simulink model "MCVT_Integration"  (R2020a)
settings: Solver=ode8  StartTime=0.0  StopTime=T_end  FixedStep=dt  RelTol=1e-3  EnableMultiTasking=on
values from neighbouring files (symbols this model uses):
  assumed_power_voltage = 1.25e2    (= 125)    [MCVT_Input_File.m]    (also in MCVT_Run_QuickCheck.m)
  battery_power_toggle = 1    [MCVT_Input_File.m]    (also in MCVT_Run_QuickCheck.m)
  battery_trickle_charge_param = 0.1    [MCVT_Input_File.m]    (also in MCVT_Run_QuickCheck.m)
  battery_trickle_leakage_param = 0.01    [MCVT_Input_File.m]    (also in MCVT_Run_QuickCheck.m)
  damage_case_id = 3    [MCVT_Input_File.m]    (also in MCVT_Run_QuickCheck.m = 1)
  dt = 1/fs    (= 0.001)    [MCVT_Run_QuickCheck.m]    (also in MCVT_Sim_Set.m)
  dt_2 = 1/fs_2    (= 0.001)    [MCVT_Run_QuickCheck.m]    (also in MCVT_Sim_Set.m)
  dt_3 = 1/fs_3    (= 0.001)    [MCVT_Run_QuickCheck.m]    (also in MCVT_Sim_Set.m)
  dt_5 = 1/fs_5    (= 0.005)    [MCVT_Run_QuickCheck.m]    (also in MCVT_Sim_Set.m)
  dt_8 = 1/fs_8    (= 0.05)    [MCVT_Run_QuickCheck.m]    (also in MCVT_Sim_Set.m)
  dt_9 = 1/fs_9    (= 0.001)    [MCVT_Run_QuickCheck.m]    (also in MCVT_Sim_Set.m)
  dust_met_duration = 3    [MCVT_Input_File.m]    (also in MCVT_Run_QuickCheck.m = 2)
  ECLSS_indoor_set_temperature = 298.15    [MCVT_Input_File.m]    (also in MCVT_Run_QuickCheck.m)
  energy_store_max_o = 150    [MCVT_Input_File.m]    (also in MCVT_Run_QuickCheck.m)
  energy_store_o = 120    [MCVT_Input_File.m]    (also in MCVT_Run_QuickCheck.m)
  fs = 1000    [MCVT_Run_QuickCheck.m]    (also in MCVT_Sim_Set.m)
  gain_charge_param = 1    [MCVT_Input_File.m]    (also in MCVT_Run_QuickCheck.m)
  ground_temperature = 373    [MCVT_Habitat_Design_Input_File.m]    (also in MCVT_Run_QuickCheck.m)
  I_max_charge = 800    [MCVT_Input_File.m]    (also in MCVT_Run_QuickCheck.m)
  I_max_discharge = 800    [MCVT_Input_File.m]    (also in MCVT_Run_QuickCheck.m)
  initial_pressure = 1e5    (= 100000)    [MCVT_Input_File.m]    (also in MCVT_Run_QuickCheck.m)
  initial_temperature = 295    [MCVT_Input_File.m]    (also in MCVT_Run_QuickCheck.m)
  interior_leak_rate = 0.00415    [MCVT_Input_File.m]    (also in MCVT_Run_QuickCheck.m)
  launch_dust_accum_start_time = 10    [MCVT_Input_File.m]    (also in MCVT_Run_QuickCheck.m = 2)
  launch_dust_accum_stop_time = 12    [MCVT_Input_File.m]    (also in MCVT_Run_QuickCheck.m = 3)
  meteorite_impact_time = 2    [MCVT_Input_File.m]    (also in MCVT_Run_QuickCheck.m = 1)
  nuclear_power_toggle = 1    [MCVT_Input_File.m]    (also in MCVT_Run_QuickCheck.m)
  print_interval = 30    [MCVT_Input_File.m]
  SOC_lower_thres = 0.5    [MCVT_Input_File.m]    (also in MCVT_Run_QuickCheck.m)
  SOC_upper_thres = 0.9    [MCVT_Input_File.m]    (also in MCVT_Run_QuickCheck.m)
  solar_power_toggle = 1    [MCVT_Input_File.m]    (also in MCVT_Run_QuickCheck.m)
  space_temperature = 4    [MCVT_Habitat_Design_Input_File.m]    (also in MCVT_Run_QuickCheck.m)
  Start_angle = 90    [MCVT_Input_File.m]    (also in MCVT_Run_QuickCheck.m)
undefined symbols (not defined in the file or in its folder): A_flow_restrictor, ACTIVITY_LOOKUP_TABLE, air, Aref, Bmap, Bmap2, Bmap3, comms, DiscIntNeverNeededParam, DmgSPL, dust_parameters, ECLSS_dust_damage_repair_time, ECLSS_heater_capacity, ECLSS_IPS_restrictor, ECLSS_IPS_setpointPressure, ECLSS_paint_damage_repair_time, ECLSS_radiatorLoop_discreteNumber, ECLSS_Vst, emptyActivity, expected_heat_add, FI, habitat, HIEM2SMM, HIEM2STM, HIEMDamageElements, impact_numbering, initial_SPLT_damage_index, input_air, input_Cond, input_CP, input_discretization, input_EV, input_Evap, input_radiatorLoop, K_ECLSS_dust_single, K_ECLSS_paint_single, K_Structure_damage_single, Kref, kW_to_W, meteoroidDustDep, mFRF, ndelays, nominalDustDep, noverlap, nuclear_toggle_gain, output_limits, panel_paint0, Ps0, reference_temperature, RepairTime_ECLSS_factor, Sensor_Location_SMM, SMM_T0, SMM_T1, SMM_T2, SMM_T3, SMM_T4, SMM_T4_0, SMM_T4_1, SMM_T4_2, solar_FDD_K ... (+73)
libraries: neural x350, simulink x39, xpcutilitieslib x7, xpcrtudplib x7, dspstat3 x1
(part 40 of 43)

== matlab function MCVT_Integration/Sys3 - Power System/Power Generation Subsystem1/Nuclear Power Generation/SP-100 Power Generation/	Power Generation/Nominal Power/Sensor Models/Cold Junction Temperature Sensing Model3/ Resistance Temperature Detector/R vs T ==
  data: R input; T output
function T = fcn(R)

T = 1.76520388802152e-09 * R^4 - 2.98990866289637e-07 * R^3 + 0.000987249006463873 * R^2 ...
    + 2.36178487086887 * R - 245.924688605077;

end

== matlab function MCVT_Integration/Sys3 - Power System/Power Generation Subsystem1/Nuclear Power Generation/SP-100 Power Generation/	Power Generation/Nominal Power/Sensor Models/Cold Junction Temperature Sensing Model3/ Resistance Temperature Detector/RTD with Self-heat Error/MATLAB Function ==
  data: I input; R input; U output
function U = fcn(I, R)

U = I * R;     % Operating current = 1mA
               % Voltage output U = I*R

== matlab function MCVT_Integration/Sys3 - Power System/Power Generation Subsystem1/Nuclear Power Generation/SP-100 Power Generation/	Power Generation/Nominal Power/Sensor Models/Cold Junction Temperature Sensing Model3/ Resistance Temperature Detector/RTD without Self-heat Error/MATLAB Function ==
  data: I input; I output; R input; U output
function [I,U]= fcn(I,R)

U = I * R;     % Operating current = 1mA
               % Voltage output U = I*R

== matlab function MCVT_Integration/Sys3 - Power System/Power Generation Subsystem1/Nuclear Power Generation/SP-100 Power Generation/	Power Generation/Nominal Power/Sensor Models/Cold Junction Temperature Sensing Model3/ Resistance Temperature Detector/Self-heat Calculation/  T vs R  ==
  data: R output; T input
function R = fcn(T)

if T >= 0
    R = 100*(1+3.9083*10^(-3)*T-5.775*10^(-7)*T^2);
else
    R = 100*(1+3.9083*10^(-3)*T-5.775*10^(-7)*T^2-4.183*10^(-12)*(T-100)*T^3);
end

== matlab function MCVT_Integration/Sys3 - Power System/Power Generation Subsystem1/Nuclear Power Generation/SP-100 Power Generation/	Power Generation/Nominal Power/Sensor Models/Cold Junction Temperature Sensing Model3/ Resistance Temperature Detector/Switch ON//OFF Control Block/Switch OFF/MATLAB Function1 ==
  data: u input; y output; Time input
function y = fcn(u,Time)

if Time>=0
    u = NaN;
end

y = u;

== matlab function MCVT_Integration/Sys3 - Power System/Power Generation Subsystem1/Nuclear Power Generation/SP-100 Power Generation/	Power Generation/Nominal Power/Sensor Models/Cold Junction Temperature Sensing Model3/ Resistance Temperature Detector/T(Celcuis) vs R ==
  data: R output; T input
function R = fcn(T)

if T >= 0
    R = 100*(1+3.9083*10^(-3)*T-5.775*10^(-7)*T^2);
else
    R = 100*(1+3.9083*10^(-3)*T-5.775*10^(-7)*T^2-4.183*10^(-12)*(T-100)*T^3);
end

== matlab function MCVT_Integration/Sys3 - Power System/Power Generation Subsystem1/Nuclear Power Generation/SP-100 Power Generation/	Power Generation/Nominal Power/Sensor Models/Cold Junction Temperature Sensing Model4/ Resistance Temperature Detector/R vs T ==
  data: R input; T output
function T = fcn(R)

T = 1.76520388802152e-09 * R^4 - 2.98990866289637e-07 * R^3 + 0.000987249006463873 * R^2 ...
    + 2.36178487086887 * R - 245.924688605077;

end

== matlab function MCVT_Integration/Sys3 - Power System/Power Generation Subsystem1/Nuclear Power Generation/SP-100 Power Generation/	Power Generation/Nominal Power/Sensor Models/Cold Junction Temperature Sensing Model4/ Resistance Temperature Detector/RTD with Self-heat Error/MATLAB Function ==
  data: I input; R input; U output
function U = fcn(I, R)

U = I * R;     % Operating current = 1mA
               % Voltage output U = I*R

== matlab function MCVT_Integration/Sys3 - Power System/Power Generation Subsystem1/Nuclear Power Generation/SP-100 Power Generation/	Power Generation/Nominal Power/Sensor Models/Cold Junction Temperature Sensing Model4/ Resistance Temperature Detector/RTD without Self-heat Error/MATLAB Function ==
  data: I input; I output; R input; U output
function [I,U]= fcn(I,R)

U = I * R;     % Operating current = 1mA
               % Voltage output U = I*R

== matlab function MCVT_Integration/Sys3 - Power System/Power Generation Subsystem1/Nuclear Power Generation/SP-100 Power Generation/	Power Generation/Nominal Power/Sensor Models/Cold Junction Temperature Sensing Model4/ Resistance Temperature Detector/Self-heat Calculation/  T vs R  ==
  data: R output; T input
function R = fcn(T)

if T >= 0
    R = 100*(1+3.9083*10^(-3)*T-5.775*10^(-7)*T^2);
else
    R = 100*(1+3.9083*10^(-3)*T-5.775*10^(-7)*T^2-4.183*10^(-12)*(T-100)*T^3);
end

== matlab function MCVT_Integration/Sys3 - Power System/Power Generation Subsystem1/Nuclear Power Generation/SP-100 Power Generation/	Power Generation/Nominal Power/Sensor Models/Cold Junction Temperature Sensing Model4/ Resistance Temperature Detector/Switch ON//OFF Control Block/Switch OFF/MATLAB Function1 ==
  data: u input; y output; Time input
function y = fcn(u,Time)

if Time>=0
    u = NaN;
end

y = u;

== matlab function MCVT_Integration/Sys3 - Power System/Power Generation Subsystem1/Nuclear Power Generation/SP-100 Power Generation/	Power Generation/Nominal Power/Sensor Models/Cold Junction Temperature Sensing Model4/ Resistance Temperature Detector/T(Celcuis) vs R ==
  data: R output; T input
function R = fcn(T)

if T >= 0
    R = 100*(1+3.9083*10^(-3)*T-5.775*10^(-7)*T^2);
else
    R = 100*(1+3.9083*10^(-3)*T-5.775*10^(-7)*T^2-4.183*10^(-12)*(T-100)*T^3);
end

== matlab function MCVT_Integration/Sys3 - Power System/Power Generation Subsystem1/Nuclear Power Generation/SP-100 Power Generation/	Power Generation/Nominal Power/Sensor Models/block/MATLAB Function2 ==
  data: y_Tf output; y_process_scale_lower input; y_Tb1 output; y_Tb2 output; y_Tc2 output
function [y_Tf, y_Tb1, y_Tb2, y_Tc2] = fcn(y_process_scale_lower)
    y_Tf = y_process_scale_lower(:,1);
    y_Tb1 = y_process_scale_lower(:,2);
    y_Tb2 = y_process_scale_lower(:,3);
    y_Tc2 = y_process_scale_lower(:,4);
end

== matlab function MCVT_Integration/Sys3 - Power System/Power Generation Subsystem1/Nuclear Power Generation/SP-100 Power Generation/	Power Generation/Nominal Power/scale [-1,1] to process value5 ==
  data: y_unitscale input; y_process_scale_lower output; output_limits input; inds input
function y_process_scale_lower = fcn(y_unitscale,output_limits, inds)
% unscale 1x1 input from [-1,1] to the process value 
unscale = @(data, l, u, inmin, inmax) (data - l) .* (inmax - inmin) ./ (u - l) + inmin;
y_process_scale_lower = [0 0 0 0];
for k=1:length(inds)
    data_min_max = output_limits(inds(k),:); %lower and upper limits for jth output
    inmin = data_min_max(1);
    inmax = data_min_max(2);
    y_process_scale_lower(k) = unscale(y_unitscale(k),-1,1,inmin,inmax);
end
end

== matlab function MCVT_Integration/Sys5 - ECLSS/Active Thermal Control System (ATCS)/Faulty Panels/MATLAB Function1 ==
  data: L_dust_k input; agent input; md input; Time input; dt input; N input; L_dust output; offset_md output; health output; timer_k input; timer output; dust_memory_k input; dust_memory output
function [L_dust, offset_md, health, timer, dust_memory] = dust_fix_with_agent(L_dust_k, agent, md, Time,  dt, N, timer_k,  dust_memory_k)
%% Documentation
% Purpose: determine panels to be cleaned and affected by natural dust
% accumulation 
% Date Created: 20 June 2020
% Date Last Modified: 27 July 2021
% Modeler Name: Jaewon Park, CJ Pan (Purdue)
% Funding Acknowledgement: Funded by the NASA RETHi Project (80NSSC19K1076)

% Version Number: MCVT v1.4

% Subsystem Connections: receives input from subsystem 9 and subsystem 6.

% INPUTS %
%   - Time: time which agents spend on cleaning the panel, unit: hr, type:
%   float
%   - L_dust_k: previous dirty panel info, [1x50], type: integer
%   - agent: agent activity, type: integer
%   - dt: timestep, unit: s, type: float 
%   - N: number of panel subsections, type: integer
%   - timer_k: recorded time duration while agent presence, unit: s, type:
%   float
%   - md: dust deposition ratio, unit: g/cm^2, type: float
%   - dust_memory_k: dust deposition ratio of previous timestep, unit:
%   g/cm^2, type: float

    
% OUTPUTS %
%   - L_dust: dirty panel info, [1x50], type: integer
%   - health: health status, ratio of dirty panels to total panels, type:
%   float
%   - dust_memory: dust deposition ratio when agent made a connection last
%   time, unit: g/cm^2, type: float
%   - offset_md: offset dust deposition ratio accounting for agents visit,
%   unit: g/cm^2, type: float

% Function Dependencies: No dependency

% No Data Depenencies
%% Generating list of panel subsections which are dirty (dust damage)
convert_hr2sec = 3600;                              % convert hours to seconds 

time_to_brush = Time*convert_hr2sec;                         % time to brush one subsection of a panel
dust_memory = dust_memory_k;                        % update dust_memory
min_dust = 0.01;                                    % for model stability (to do) investigating other method which does not require this hard coding 
max_dust = 0.1;                                     % for model stability (to do) ''
n_max_natural_dust = 15 ;                           % maximum number of subsection affected by natural acumulaiton.(no impact)(To do) update needed as we move on from 60s simulation limit
n_panels_natural = round(n_max_natural_dust*((md-dust_memory_k)-min_dust)/max_dust);    % number of subsections that should be affected by the dust based on dust parameter
if n_panels_natural < 0             
    n_panels_natural = 0;                           % in case it turns out to be a negative number. Force non negative
elseif n_panels_natural > n_max_natural_dust
        n_panels_natural = n_max_natural_dust;      % saftey mechanism
end

%disp(n_panels_natural)
if L_dust_k == 0                                    % if there is no panel to clean
   
    if n_panels_natural == 0 
        L_dust = L_dust_k;                              % output the same result 
    else
        L_dust_k(end) = randToAdd(L_dust_k,N);        % see the function below
        L_dust = sort(L_dust_k,'descend');         % sort things so that you have zeros towards the end
    end
    health = 0;                                     % health state is healthy so 0
    timer = 0;                                      % reset timer// If some dust was added in the 'if' statement, the status will be reflected at next dt
    
else                                                % if there is some panels to clean
    Damaged_panel = L_dust_k(L_dust_k ~= 0);        % get rid of element with id=0 (These were placeholder values that just maintains the size of stream consistent) 
    n_panel_to_fix = length(Damaged_panel);         % count the number of subsections of the panel to be cleaned
    
    if agent == 1                                   % if agent is present
        dust_memory = md;                           % this is going to be offset value for next iteration 
        if timer_k > time_to_brush                  % if elasped time is greater than tiem to brush time
            timer = 0;                              % reset timer
            L_dust_k(n_panel_to_fix:end)=0;         % mark panels zero so that you have one less panel to clean
        else                                        % if elasped time is not enough
            timer = timer_k + dt;                   % add time
        end
    else                                            % if there is no agent
        timer = 0;                                   % reset timer
        if n_panel_to_fix < n_panels_natural         % if dust parameter calls for more dust to be present than the current value,
            L_dust_k(end) = randToAdd(L_dust_k,N);     % see the function below
            L_dust_k = sort(L_dust_k,'descend');        % sort things so that you have zeros towards the end (little bit different than before because of next two lines)
        end
    end
    L_dust = L_dust_k;                              % update the panel data
    health = n_panel_to_fix/N ;                     % update health state, this gives a ratio of panels to be cleaned over total number of subsections
end

offset_md = md-dust_memory_k;

if offset_md<0
    offset_md=0;
end

end

%% randomly assign dirty panel indices 
function num = randToAdd(L_dust,N)
    while 1                                         % run forever
        temp = randi(N);                            % get a random integer
        if isempty(find(L_dust == temp,1))        % see if this integer is not already in there
            num = temp;                             % get out of the loop, you figured out what to add next
            break;
        end
    end
end

== matlab function MCVT_Integration/Sys3 - Power System/Power Generation Subsystem1/Nuclear Power Generation/SP-100 Power Generation/	Power Generation/Power Generation/Heat Rejection1/MATLAB Function ==
  data: dust_ratio input; HRF output
function HRF = fcn(dust_ratio)
HRF = 1 - (dust_ratio/60);
end

== matlab function MCVT_Integration/Sys3 - Power System/Power Generation Subsystem1/Nuclear Power Generation/SP-100 Power Generation/	Power Generation/Power Generation/MATLAB Function3 ==
  data: hrf_in input; hrf_out output
function hrf_out = fcn(hrf_in)
if hrf_in < 0
    hrf_out = 0;
else
    hrf_out = hrf_in;
end
end

== matlab function MCVT_Integration/Sys3 - Power System/Power Generation Subsystem1/Nuclear Power Generation/SP-100 Power Generation/	Power Generation/Power Generation/Secondary Loop + Stirling Loop/MATLAB Function1 ==
  data: u input; Tc2_lower output
function Tc2_lower = fcn(u)
Tc2_lower = u(:,4);
end

== matlab function MCVT_Integration/Sys3 - Power System/Power Generation Subsystem1/Nuclear Power Generation/SP-100 Power Generation/	Power Generation/Power Generation/Upoer and Lowew Dust ==
  data: hrf input; dust_up output; dust_low output
function [dust_up,dust_low] = fcn(hrf)
all_levels = 0:5:100; hrf = hrf*100;
dust_low = 0;
for i = 1:20
    if hrf >= all_levels(i)
        dust_low = i;
    end
end
dust_up = dust_low + 1;
end

== matlab function MCVT_Integration/Sys3 - Power System/Power Generation Subsystem1/Nuclear Power Generation/SP-100 Power Generation/	Power Generation/Power Generation/scale [-1,1] to process value1 ==
  data: y_unitscale input; y_process_scale_upper output; output_limits input; inds input
function y_process_scale_upper = fcn(y_unitscale,output_limits, inds)
% unscale 1x1 input from [-1,1] to the process value 
unscale = @(data, l, u, inmin, inmax) (data - l) .* (inmax - inmin) ./ (u - l) + inmin;
y_process_scale_upper = [0 0 0 0];
for k=1:length(inds)
    data_min_max = output_limits(inds(k),:); %lower and upper limits for jth output
    inmin = data_min_max(1);
    inmax = data_min_max(2);
    y_process_scale_upper(k) = unscale(y_unitscale(k),-1,1,inmin,inmax);
end
end

== matlab function MCVT_Integration/Sys3 - Power System/Power Generation Subsystem1/Nuclear Power Generation/SP-100 Power Generation/	Power Generation/Power Generation/scale [-1,1] to process value5 ==
  data: y_unitscale input; y_process_scale_lower output; output_limits input; inds input
function y_process_scale_lower = fcn(y_unitscale,output_limits, inds)
% unscale 1x1 input from [-1,1] to the process value 
unscale = @(data, l, u, inmin, inmax) (data - l) .* (inmax - inmin) ./ (u - l) + inmin;
y_process_scale_lower = [0 0 0 0];
for k=1:length(inds)
    data_min_max = output_limits(inds(k),:); %lower and upper limits for jth output
    inmin = data_min_max(1);
    inmax = data_min_max(2);
    y_process_scale_lower(k) = unscale(y_unitscale(k),-1,1,inmin,inmax);
end
end

== matlab function MCVT_Integration/Sys3 - Power System/Power Generation Subsystem1/Nuclear Power Generation/SP-100 Power Generation/	Power Generation/Power Generation/scale process value to [-1,1]2 ==
  data: u input; y output; output_limits input; ind input
function y = fcn(u,output_limits,ind)
% Converts an input row vector to [-1,1] 
% example: u = [simOut.sec_flux.data, simOut.row.data, ...
% input_limits = [1000, 1400; 900, 1200; ...]
scale = @(data, l, u, inmin, inmax) l + ((data-inmin)./(inmax-inmin)).*(u-l);
j = ind;
data_min_max = output_limits(j,:); %lower and upper limits for jth output
inmin = data_min_max(1);
inmax = data_min_max(2);
y = scale(u,-1,1,inmin,inmax);

== matlab function MCVT_Integration/Sys3 - Power System/Power Generation Subsystem1/Nuclear Power Generation/SP-100 Power Generation/	Power Generation/Sensor Models/Cold Junction Temperature Sensing Model/ Resistance Temperature Detector/R vs T ==
  data: R input; T output
function T = fcn(R)

T = 1.76520388802152e-09 * R^4 - 2.98990866289637e-07 * R^3 + 0.000987249006463873 * R^2 ...
    + 2.36178487086887 * R - 245.924688605077;

end

== matlab function MCVT_Integration/Sys3 - Power System/Power Generation Subsystem1/Nuclear Power Generation/SP-100 Power Generation/	Power Generation/Sensor Models/Cold Junction Temperature Sensing Model/ Resistance Temperature Detector/RTD with Self-heat Error/MATLAB Function ==
  data: I input; R input; U output
function U = fcn(I, R)

U = I * R;     % Operating current = 1mA
               % Voltage output U = I*R

== matlab function MCVT_Integration/Sys3 - Power System/Power Generation Subsystem1/Nuclear Power Generation/SP-100 Power Generation/	Power Generation/Sensor Models/Cold Junction Temperature Sensing Model/ Resistance Temperature Detector/RTD without Self-heat Error/MATLAB Function ==
  data: I input; I output; R input; U output
function [I,U]= fcn(I,R)

U = I * R;     % Operating current = 1mA
               % Voltage output U = I*R

== matlab function MCVT_Integration/Sys3 - Power System/Power Generation Subsystem1/Nuclear Power Generation/SP-100 Power Generation/	Power Generation/Sensor Models/Cold Junction Temperature Sensing Model/ Resistance Temperature Detector/Self-heat Calculation/  T vs R  ==
  data: R output; T input
function R = fcn(T)

if T >= 0
    R = 100*(1+3.9083*10^(-3)*T-5.775*10^(-7)*T^2);
else
    R = 100*(1+3.9083*10^(-3)*T-5.775*10^(-7)*T^2-4.183*10^(-12)*(T-100)*T^3);
end

== matlab function MCVT_Integration/Sys3 - Power System/Power Generation Subsystem1/Nuclear Power Generation/SP-100 Power Generation/	Power Generation/Sensor Models/Cold Junction Temperature Sensing Model/ Resistance Temperature Detector/Switch ON//OFF Control Block/Switch OFF/MATLAB Function1 ==
  data: u input; y output; Time input
function y = fcn(u,Time)

if Time>=0
    u = NaN;
end

y = u;

== matlab function MCVT_Integration/Sys3 - Power System/Power Generation Subsystem1/Nuclear Power Generation/SP-100 Power Generation/	Power Generation/Sensor Models/Cold Junction Temperature Sensing Model/ Resistance Temperature Detector/T(Celcuis) vs R ==
  data: R output; T input
function R = fcn(T)

if T >= 0
    R = 100*(1+3.9083*10^(-3)*T-5.775*10^(-7)*T^2);
else
    R = 100*(1+3.9083*10^(-3)*T-5.775*10^(-7)*T^2-4.183*10^(-12)*(T-100)*T^3);
end

== matlab function MCVT_Integration/Sys3 - Power System/Power Generation Subsystem1/Nuclear Power Generation/SP-100 Power Generation/	Power Generation/Sensor Models/Cold Junction Temperature Sensing Model1/ Resistance Temperature Detector/R vs T ==
  data: R input; T output
function T = fcn(R)

T = 1.76520388802152e-09 * R^4 - 2.98990866289637e-07 * R^3 + 0.000987249006463873 * R^2 ...
    + 2.36178487086887 * R - 245.924688605077;

end

== matlab function MCVT_Integration/Sys3 - Power System/Power Generation Subsystem1/Nuclear Power Generation/SP-100 Power Generation/	Power Generation/Sensor Models/Cold Junction Temperature Sensing Model1/ Resistance Temperature Detector/RTD with Self-heat Error/MATLAB Function ==
  data: I input; R input; U output
function U = fcn(I, R)

U = I * R;     % Operating current = 1mA
               % Voltage output U = I*R

== matlab function MCVT_Integration/Sys3 - Power System/Power Generation Subsystem1/Nuclear Power Generation/SP-100 Power Generation/	Power Generation/Sensor Models/Cold Junction Temperature Sensing Model1/ Resistance Temperature Detector/RTD without Self-heat Error/MATLAB Function ==
  data: I input; I output; R input; U output
function [I,U]= fcn(I,R)

U = I * R;     % Operating current = 1mA
               % Voltage output U = I*R

== matlab function MCVT_Integration/Sys3 - Power System/Power Generation Subsystem1/Nuclear Power Generation/SP-100 Power Generation/	Power Generation/Sensor Models/Cold Junction Temperature Sensing Model1/ Resistance Temperature Detector/Self-heat Calculation/  T vs R  ==
  data: R output; T input
function R = fcn(T)

if T >= 0
    R = 100*(1+3.9083*10^(-3)*T-5.775*10^(-7)*T^2);
else
    R = 100*(1+3.9083*10^(-3)*T-5.775*10^(-7)*T^2-4.183*10^(-12)*(T-100)*T^3);
end

== matlab function MCVT_Integration/Sys3 - Power System/Power Generation Subsystem1/Nuclear Power Generation/SP-100 Power Generation/	Power Generation/Sensor Models/Cold Junction Temperature Sensing Model1/ Resistance Temperature Detector/Switch ON//OFF Control Block/Switch OFF/MATLAB Function1 ==
  data: u input; y output; Time input
function y = fcn(u,Time)

if Time>=0
    u = NaN;
end

y = u;

== matlab function MCVT_Integration/Sys3 - Power System/Power Generation Subsystem1/Nuclear Power Generation/SP-100 Power Generation/	Power Generation/Sensor Models/Cold Junction Temperature Sensing Model1/ Resistance Temperature Detector/T(Celcuis) vs R ==
  data: R output; T input
function R = fcn(T)

if T >= 0
    R = 100*(1+3.9083*10^(-3)*T-5.775*10^(-7)*T^2);
else
    R = 100*(1+3.9083*10^(-3)*T-5.775*10^(-7)*T^2-4.183*10^(-12)*(T-100)*T^3);
end

== matlab function MCVT_Integration/Sys3 - Power System/Power Generation Subsystem1/Nuclear Power Generation/SP-100 Power Generation/	Power Generation/Sensor Models/Cold Junction Temperature Sensing Model2/ Resistance Temperature Detector/R vs T ==
  data: R input; T output
function T = fcn(R)

T = 1.76520388802152e-09 * R^4 - 2.98990866289637e-07 * R^3 + 0.000987249006463873 * R^2 ...
    + 2.36178487086887 * R - 245.924688605077;

end

== matlab function MCVT_Integration/Sys3 - Power System/Power Generation Subsystem1/Nuclear Power Generation/SP-100 Power Generation/	Power Generation/Sensor Models/Cold Junction Temperature Sensing Model2/ Resistance Temperature Detector/RTD with Self-heat Error/MATLAB Function ==
  data: I input; R input; U output
function U = fcn(I, R)

U = I * R;     % Operating current = 1mA
               % Voltage output U = I*R

== matlab function MCVT_Integration/Sys3 - Power System/Power Generation Subsystem1/Nuclear Power Generation/SP-100 Power Generation/	Power Generation/Sensor Models/Cold Junction Temperature Sensing Model2/ Resistance Temperature Detector/RTD without Self-heat Error/MATLAB Function ==
  data: I input; I output; R input; U output
function [I,U]= fcn(I,R)

U = I * R;     % Operating current = 1mA
               % Voltage output U = I*R

== matlab function MCVT_Integration/Sys3 - Power System/Power Generation Subsystem1/Nuclear Power Generation/SP-100 Power Generation/	Power Generation/Sensor Models/Cold Junction Temperature Sensing Model2/ Resistance Temperature Detector/Self-heat Calculation/  T vs R  ==
  data: R output; T input
function R = fcn(T)

if T >= 0
    R = 100*(1+3.9083*10^(-3)*T-5.775*10^(-7)*T^2);
else
    R = 100*(1+3.9083*10^(-3)*T-5.775*10^(-7)*T^2-4.183*10^(-12)*(T-100)*T^3);
end

== matlab function MCVT_Integration/Sys9 - Exterior Environment/Exterior Environment/MATLAB Function ==
  data: Ag_x output; t input; Ag_y output; Magnitude input
function [Ag_x,Ag_y]   = fcn(t,Magnitude)

dw = 1; %frequency resolution
D = 0.22*1e6; %m2/s
Q = 8000;
ro = 1740; %kg/m3
w = 0.5*2*pi:dw:20*pi;
N = length(w);
alpha = unifrnd(0,2*pi);
R = unifrnd(10e3,10e3);


E = 10^(Magnitude*2.4-1.2);
energy = 0; di = 0.01;
for i = 0.5*2*pi:di:20*pi
    energy = energy + exp(-0.5*((i-6*pi)/2/pi).^2)*di;
end
K = E/energy;
E0 = K*exp(-0.5*((w-6*pi)/2/pi).^2);

Saa = (w.^2.*E0)/(ro*(4*pi*D*t)^(1.5)).*exp(-R^2./(4*D.*t)-w.*t/Q);

%
phi = 2*pi*rand(1,N);

Ag = 0;

for i = 1:N
    Ag = Ag + cos(w(i)*t-phi(i))*sqrt(2*Saa(i)*dw);
end

Ag_x = Ag*cos(alpha);
Ag_y = Ag*sin(alpha);

if isnan(Ag_x) || isnan(Ag_y)
    Ag_x=0;
    Ag_y=0;
end

== matlab function MCVT_Integration/Sys3 - Power System/Power Generation Subsystem1/Nuclear Power Generation/SP-100 Power Generation/	Power Generation/Sensor Models/Cold Junction Temperature Sensing Model2/ Resistance Temperature Detector/Switch ON//OFF Control Block/Switch OFF/MATLAB Function1 ==
  data: u input; y output; Time input
function y = fcn(u,Time)

if Time>=0
    u = NaN;
end

y = u;

== matlab function MCVT_Integration/Sys3 - Power System/Power Generation Subsystem1/Nuclear Power Generation/SP-100 Power Generation/	Power Generation/Sensor Models/Cold Junction Temperature Sensing Model2/ Resistance Temperature Detector/T(Celcuis) vs R ==
  data: R output; T input
function R = fcn(T)

if T >= 0
    R = 100*(1+3.9083*10^(-3)*T-5.775*10^(-7)*T^2);
else
    R = 100*(1+3.9083*10^(-3)*T-5.775*10^(-7)*T^2-4.183*10^(-12)*(T-100)*T^3);
end

== matlab function MCVT_Integration/Sys3 - Power System/Power Generation Subsystem1/Nuclear Power Generation/SP-100 Power Generation/	Power Generation/Sensor Models/Cold Junction Temperature Sensing Model3/ Resistance Temperature Detector/R vs T ==
  data: R input; T output
function T = fcn(R)

T = 1.76520388802152e-09 * R^4 - 2.98990866289637e-07 * R^3 + 0.000987249006463873 * R^2 ...
    + 2.36178487086887 * R - 245.924688605077;

end

== matlab function MCVT_Integration/Sys3 - Power System/Power Generation Subsystem1/Nuclear Power Generation/SP-100 Power Generation/	Power Generation/Sensor Models/Cold Junction Temperature Sensing Model3/ Resistance Temperature Detector/RTD with Self-heat Error/MATLAB Function ==
  data: I input; R input; U output
function U = fcn(I, R)

U = I * R;     % Operating current = 1mA
               % Voltage output U = I*R

== matlab function MCVT_Integration/Sys3 - Power System/Power Generation Subsystem1/Nuclear Power Generation/SP-100 Power Generation/	Power Generation/Sensor Models/Cold Junction Temperature Sensing Model3/ Resistance Temperature Detector/RTD without Self-heat Error/MATLAB Function ==
  data: I input; I output; R input; U output
function [I,U]= fcn(I,R)

U = I * R;     % Operating current = 1mA
               % Voltage output U = I*R

== matlab function MCVT_Integration/Sys3 - Power System/Power Generation Subsystem1/Nuclear Power Generation/SP-100 Power Generation/	Power Generation/Sensor Models/Cold Junction Temperature Sensing Model3/ Resistance Temperature Detector/Self-heat Calculation/  T vs R  ==
  data: R output; T input
function R = fcn(T)

if T >= 0
    R = 100*(1+3.9083*10^(-3)*T-5.775*10^(-7)*T^2);
else
    R = 100*(1+3.9083*10^(-3)*T-5.775*10^(-7)*T^2-4.183*10^(-12)*(T-100)*T^3);
end

== matlab function MCVT_Integration/Sys3 - Power System/Power Generation Subsystem1/Nuclear Power Generation/SP-100 Power Generation/	Power Generation/Sensor Models/Cold Junction Temperature Sensing Model3/ Resistance Temperature Detector/Switch ON//OFF Control Block/Switch OFF/MATLAB Function1 ==
  data: u input; y output; Time input
function y = fcn(u,Time)

if Time>=0
    u = NaN;
end

y = u;

== matlab function MCVT_Integration/Sys9 - Exterior Environment/Exterior Environment/Determine_Damage_Case ==
  data: FI_index output; dmg_caseo output; t input; dmg_case input; mtime input; countp input; FI_tend input; count output; FI parameter; FI_tend output
function [FI_index,dmg_caseo,count,FI_tend]  = fcn(t,dmg_case,mtime,countp,FI_tend, FI)

count=0;
dmg_caseo=0;

FI_index=[0 0];

if t >= mtime && FI_tend == 0
%     disp('size of FI')
%     size(FI,1)
    dmg_caseo=dmg_case;
    if countp<=size(FI,1)-1
        count=countp+1;
        if count==1
            disp('        Meteorite Impact Happens');
        end        
        FI_index=[1 count];
    elseif countp==size(FI,1)
        count=0;
        FI_tend=1;
        dmg_caseo=0;
    end
    
end


end

== matlab function MCVT_Integration/Sys9 - Exterior Environment/Exterior Environment/Impact_Location ==
  data: FI_Loc output; meteorite_impact_location_xyz parameter
function FI_Loc  = impact_location(meteorite_impact_location_xyz)


FI_Loc=meteorite_impact_location_xyz;

    
end

== matlab function MCVT_Integration/Sys3 - Power System/Power Generation Subsystem1/Nuclear Power Generation/SP-100 Power Generation/	Power Generation/Sensor Models/Cold Junction Temperature Sensing Model3/ Resistance Temperature Detector/T(Celcuis) vs R ==
  data: R output; T input
function R = fcn(T)

if T >= 0
    R = 100*(1+3.9083*10^(-3)*T-5.775*10^(-7)*T^2);
else
    R = 100*(1+3.9083*10^(-3)*T-5.775*10^(-7)*T^2-4.183*10^(-12)*(T-100)*T^3);
end

== matlab function MCVT_Integration/Sys8 - Interior Environment/Habitat Interior Environment Model/HIEM2SMM_mapping ==
  data: HIEM_WallPress input; HIEM_WallPress_out output; HIEM2SMM input; b input
function HIEM_WallPress_out = HIEM2SMM_mapping(HIEM_WallPress, HIEM2SMM, b)

% This function block maps the calculated pressure to the mesh in Sys2 using the
% script values initialized by "Sys8_in_env.m"

P1 = HIEM_WallPress;

for i=1:length(HIEM2SMM)

    HIEM2SMM(i) = P1(b(i));
end

HIEM_WallPress_out = HIEM2SMM;

end

== matlab function MCVT_Integration/Sys 6 - Health Management and Agents/ Agent System/Agent Module/Action Scheduler/module1 ==
  data: program input; meta_pgm output; todo_pgm output; nValidActions output; EMPTY parameter
%         #=================================================#
%         #    Code developed for the RETH-Institute        #
%         #    By: Murali Krishnan Rajasekharan Pillai      #
%         #    PhD Student @ Purdue University              #
%         #    Funded by: NASA                              #
%         #    Last Modified: July 29, 2021                 #
%         #=================================================#
%     
% Activity Pre-processor
function [meta_pgm, todo_pgm, nValidActions] = activityPreprocessor(program, EMPTY)
%       Purpose: This function processes the `pgm` command from the Memory module to
%       derive the appropriate inputs to the Stateflow module down-stream
%     
%       Input:
%       program     :   2-D float array
%                       Program of size [(NUM_MAX_ACTIONS + 1) x NUM_MAX_ACT_PARAMS]
%     
%       Output:
%       meta_pgm    :   1-D float array
%                       The meta-data of the program [1 x NUM_MAX_ACT_PARAMS]
%       todo_pgm    :   2D float array
%                       The TODO information [NUM_MAX_ACTIONS x NUM_MAX_ACT_PARAMS]
%       nValidActions:  float
%                       The number of valid actions in the current TODO
    UNDF = EMPTY;
    [nR, nC] = size(program);
    EMPTY_PGM = EMPTY * ones(nR, nC);
    isEmpty = isequal(program, EMPTY_PGM);
    meta_pgm = program(1,1:end);
    todo_pgm = program(2:end,1:end);
    
    if ~isEmpty
        nValidActions = countActions(program);
    else
        nValidActions = 0;        
    end
    
    
    
end

%     Purpose: Count the number of valid actions in the program
function countValActs = countActions(valPgm)

%     Input:
%     valPgm        :   2D float arrray
%                       A valid program
%     
%     Output:
%     countValActs  :   float
%                       Count of the valid actions in the Activity Program
    [nR, ~] = size(valPgm);
    countValActs = 0;
    for i=2:nR
        if valPgm(i,1) > 0
            countValActs = countValActs + 1;
        end
    end
end

== matlab function MCVT_Integration/Sys8 - Interior Environment/Habitat Interior Environment Model/HIEM2STM_mapping ==
  data: HIEM_WallTemp input; HIEM_WallTemp_out output; HIEM2STM input; b input
function HIEM_WallTemp_out = HIEM2STM_mapping(HIEM_WallTemp, HIEM2STM, b)

% This function block maps the air temperature adjacent to the wall to the 
% mesh in Sys2 using the script values initialized by "Sys8_in_env.m"

T1 = HIEM_WallTemp;

%% mapping to STM
for i=1:length(HIEM2STM)
    HIEM2STM(i) = T1(b(i));
end

HIEM_WallTemp_out = HIEM2STM;

end

== matlab function MCVT_Integration/Sys8 - Interior Environment/Habitat Interior Environment Model/Humans2HIEM ==
  data: HumanHeat output; HumanEnergy input; r input; HumansElement output; theta input; air_specific_heat input
function [HumanHeat,HumansElement] = Humans2HIEM(HumanEnergy,r,theta,air_specific_heat)

% This function block maps the heat given by the Human Agent model to the
% closest Sys8 element, as well as converts that heat value to net
% temperature change.

HumanHeat = HumanEnergy/(air_specific_heat * 0.82 * 1); %J/(J/kg-K * kg/m^3 * m^3), Output is Kelvin

hx = [r * cos(theta)];
hy = [r * sin(theta)];
hz = [1];

% HIEM_EN112
Xex_HIEM = [-1.031321213,-0.374273315,-0.992938884,-0.374180816,-0.372558691,-0.400335357,-0.427111223,-1.000331663,-1.106535197,-1.191996857,-1.70044367,-1.948610396,-1.560300425,-1.771134109,-1.352136761,-1.481280162,-0.341369473,-0.362746552,-0.392023116,-0.33329244,-0.35387215,-0.382010765,-0.963476307,-1.069203303,-1.16669199,-0.911662013,-0.998376087,-1.084986158,1.03456854,0.375939019,0.99351266,0.374227405,0.345859639,0.366851881,0.39294786,0.969574949,1.072633846,1.165673426,0.334103949,0.354160473,0.379702896,0.912960312,0.997980013,1.079399883,1.947061791,1.770781874,1.483332395,1.697034776,1.558642045,1.35183978,0.371551521,0.399117634,0.424516231,0.997977487,1.10331601,1.186484576,-0.425756931,-1.190424413,-0.397799045,-1.104461506,-0.371766724,-1.001441203,-1.167114742,-0.391985722,-1.084650122,-0.381620027,-1.066377573,-0.361150034,-0.997034394,-0.352806278,-0.955304377,-0.337869339,-0.910463326,-0.332383938,-1.700452521,-1.949282765,-1.55997865,-1.769530952,-1.354277894,-1.477576913,-0.373312496,-0.375300467,-1.028932668,-0.996530293,1.165590509,0.391487852,1.066200681,0.360920005,0.956165851,0.3378582,1.078293293,0.378812254,0.995987893,0.352353558,0.912286789,0.332709774,0.372269094,1.030100525,0.374632142,1.000348204,1.481988551,1.771445603,1.948271274,1.361015993,1.5612406,1.696887925,0.424302414,0.399514876,0.373381779,1.186416775,1.105524653,1.005382762]';
Yex_HIEM = [-1.896619544,-2.070022344,-1.730274305,-1.890119806,-1.547566921,-1.007270664,-0.348688915,-1.443086981,-0.954992748,-0.332794987,-0.333890967,-0.350082532,-0.958206302,-1.011868208,-1.448404848,-1.555789769,-1.505951315,-0.926660032,-0.313346088,-1.44935906,-0.927602463,-0.318657048,-1.456514179,-0.931408576,-0.319526263,-1.380113974,-0.904216781,-0.313053921,-1.892578855,-2.068297176,-1.728676528,-1.88969393,-1.501782491,-0.922870837,-0.31209708,-1.448607133,-0.925002456,-0.317601398,-1.447316496,-0.925798676,-0.318030156,-1.376199185,-0.901123493,-0.312110454,-0.348009765,-1.007706851,-1.551385658,-0.332028188,-0.955485739,-1.446375163,-1.548300117,-1.007933728,-0.348882563,-1.443863705,-0.955480106,-0.332794316,0.34933348,0.335112684,1.014144288,0.966822826,1.55975646,1.460714595,0.32154353,0.314913034,0.315654695,0.320002414,0.938042611,0.931599483,0.912233099,0.934509077,1.463379382,1.511640265,1.38999021,1.460426316,0.337151855,0.351754859,0.969804898,1.018518254,1.460975513,1.561668336,2.072217165,1.898076788,1.898441329,1.7394775,0.319927014,0.314217046,0.933963068,0.92990771,1.459130809,1.510020896,0.311889447,0.31865371,0.902847178,0.930607238,1.379176586,1.455771476,2.072217821,1.895183415,1.894294515,1.725711226,1.552737074,1.009884694,0.348793991,1.439209448,0.952546873,0.331675895,0.349101424,1.009676398,1.551737698,0.332365535,0.953730473,1.43986103]';
Zex_HIEM = [0.378484965,0.378428213,1.006702021,1.041591502,1.490418389,1.773482054,1.947170794,1.365422666,1.563938171,1.698159381,1.188140876,0.424950562,1.108522699,0.40107356,1.00952109,0.375864111,0.346455924,0.365264572,0.391789347,0.972813166,1.072410084,1.164715707,0.335936144,0.354213938,0.37917497,0.919206738,0.999939606,1.079653225,0.379221477,0.378805414,1.00707347,1.04151088,0.347319603,0.366418369,0.393582687,0.337173008,0.355887264,0.382649548,0.973676129,1.073809437,1.167331114,0.92056904,1.002244652,1.084778272,0.426776543,0.402129591,0.376874521,1.191380442,1.111092299,1.011334583,1.48968105,1.77341774,1.948005556,1.36548841,1.565387428,1.700407729,1.94848703,1.698157148,1.769610064,1.555096732,1.475874706,1.343885124,0.378415957,0.392196558,1.078499198,1.16603733,0.351115279,0.362866834,0.992961251,1.067554654,0.330540888,0.338987462,0.905279957,0.956018664,1.186165213,0.423279956,1.097254695,0.395492017,0.98987832,0.368297823,0.373084195,1.026892028,0.3726063,0.988125019,0.383299619,0.394470513,0.355651997,0.36495845,0.334620379,0.340723127,1.085879088,1.16933763,1.00156086,1.071150363,0.915564246,0.960227891,0.373231605,0.375509642,1.031181602,1.001709141,0.374881521,0.402066179,0.427447401,1.008566217,1.110702896,1.192251452,1.949017555,1.772040279,1.482536121,1.700606466,1.564400987,1.362650737]';

Humansxyz = [hx hy hz];
HIEMxyz = [Xex_HIEM Yex_HIEM Zex_HIEM];

HIEMElements = 1;

%% Humans mapping to HIEM
Humans2HIEM = zeros(size(hx,1),1);

for i=1:size(hx,1)
    dxyz = nan(size(Xex_HIEM,1),1);
    for j=1:size(Xex_HIEM,1)
        dxyz_Tmp = [HIEMxyz(j,:) ; Humansxyz(i,:)];
        dxyz(j,1) = pdist(dxyz_Tmp,'euclidean');
    end
    [a,b] = min(dxyz);
    Humans2HIEM(i) = b;
end

HumansElement = Humans2HIEM;

end

== matlab function MCVT_Integration/Sys8 - Interior Environment/Habitat Interior Environment Model/K2C ==
  data: K input; C output
function C = TempConversionK2C(K)

C = K - 273.15;

end

== matlab function MCVT_Integration/Sys8 - Interior Environment/Habitat Interior Environment Model/Sys8_Time_Function ==
  data: AnalysisTime input; Time output; Denom input; dt input
function Time  = Time_Function(AnalysisTime,Denom,dt)

AnalysisTime = AnalysisTime*Denom; %Denom is 1, so this transfers model time to a useable variable

if AnalysisTime == 0
    Time = -1;
else 
    if AnalysisTime == dt 
        Time = 1; % identify the first time step
    else
        Time = 2; % identify additional time steps except the first time step
    end

end

== matlab function MCVT_Integration/Sys8 - Interior Environment/Habitat Interior Environment Model/Pressure Sensor Input ==
  data: Power input; AirPressure input; PressureAtSensor output; T input
function PressureAtSensor = PressureSensorInput(Power,AirPressure, T)

% This is a placeholder function block meant to simulate measurement of
% pressure by a pressure sensor. It is recommended that this be replaced by
% a pressure sensor model that reflects accurate sensor behavior in future 
% model iterations.

if Power == 0 && T>1
    PressureAtSensor = NaN;
else
    if length(AirPressure)<55
        PressureAtSensor = AirPressure(1);
    else
        PressureAtSensor = AirPressure(55);
    end
end

end

== matlab function MCVT_Integration/Sys8 - Interior Environment/Habitat Interior Environment Model/STM2HIEM_mapping ==
  data: STM_WallTemp input; HIEM_WallTemp output; b input; Temp2HIEM input
function HIEM_WallTemp= STM2HIEM_mapping(STM_WallTemp, b, Temp2HIEM)

% This function block maps the wall temperature given by Sys2 using the
% script values initialized by "Sys8_in_env.m"

T1 = STM_WallTemp;

for i=1:length(Temp2HIEM)
    Temp2HIEM(i)=T1(b(i));
end

HIEM_WallTemp = Temp2HIEM;

end

== matlab function MCVT_Integration/Sys8 - Interior Environment/Habitat Interior Environment Model/Temperature Sensors/ Resistance Temperature Detector/Power Consumption ==
  data: Supply input; kWUsage1 output; Switch input
function kWUsage1 = Power_Consumption(Supply,Switch)
if Switch >= 1
    Voltage = 5.5; %Volts
    Current = 0.000001; %10 microAmperes
    kWUsage1 = ones(1,1);
    
    kWUsage1 = (Voltage * Current)/1000;
    
    if kWUsage1 >= Supply
        kWUsage1 = Supply;
    end
else
    kWUsage1 = 0;
end
end

== matlab function MCVT_Integration/Sys8 - Interior Environment/Habitat Interior Environment Model/Temperature Sensors/ Resistance Temperature Detector/R vs T ==
  data: R input; T output
function T = fcn(R)

T = 1.76520388802152e-09 * R^4 - 2.98990866289637e-07 * R^3 + 0.000987249006463873 * R^2 ...
    + 2.36178487086887 * R - 245.924688605077;

end

== matlab function MCVT_Integration/Sys8 - Interior Environment/Habitat Interior Environment Model/Temperature Sensors/ Resistance Temperature Detector/RTD with Self-heat Error/MATLAB Function ==
  data: I input; R input; U output
function U = fcn(I, R)

U = I * R;     % Operating current = 1mA
               % Voltage output U = I*R

== matlab function MCVT_Integration/Sys8 - Interior Environment/Habitat Interior Environment Model/Temperature Sensors/ Resistance Temperature Detector/RTD without Self-heat Error/MATLAB Function ==
  data: I input; I output; R input; U output
function [I,U]= fcn(I,R)

U = I * R;     % Operating current = 1mA
               % Voltage output U = I*R

== matlab function MCVT_Integration/Sys8 - Interior Environment/Habitat Interior Environment Model/Temperature Sensors/ Resistance Temperature Detector/Self-heat Calculation/  T vs R  ==
  data: R output; T input
function R = fcn(T)

if T >= 0
    R = 100*(1+3.9083*10^(-3)*T-5.775*10^(-7)*T^2);
else
    R = 100*(1+3.9083*10^(-3)*T-5.775*10^(-7)*T^2-4.183*10^(-12)*(T-100)*T^3);
end

== matlab function MCVT_Integration/Sys8 - Interior Environment/Habitat Interior Environment Model/Temperature Sensors/ Resistance Temperature Detector/Switch ON//OFF Control Block/Switch OFF/MATLAB Function1 ==
  data: u input; y output; Time input
function y = fcn(u,Time)

if Time>=0
    u = NaN;
end

y = u;

== matlab function MCVT_Integration/Sys2 - Structural System/Sys2_Structural_System/Structural Thermal Model 2.2/STM Temperature Sensors/ Resistance Temperature Detector/R vs T ==
  data: R input; T output
function T = fcn(R)

T = 1.76520388802152e-09 * R^4 - 2.98990866289637e-07 * R^3 + 0.000987249006463873 * R^2 ...
    + 2.36178487086887 * R - 245.924688605077;

end

== matlab function MCVT_Integration/Sys8 - Interior Environment/Habitat Interior Environment Model/Temperature Sensors/ Resistance Temperature Detector/T vs R ==
  data: R output; T input
function R = fcn(T)

if T >= 0
    R = 100*(1+3.9083*10^(-3)*T-5.775*10^(-7)*T^2);
else
    R = 100*(1+3.9083*10^(-3)*T-5.775*10^(-7)*T^2-4.183*10^(-12)*(T-100)*T^3);
end

== matlab function MCVT_Integration/Sys8 - Interior Environment/Habitat Interior Environment Model/Temperature Sensors/ Resistance Temperature Detector1/Power Consumption ==
  data: Supply input; kWUsage2 output; Switch input
function kWUsage2 = Power_Consumption(Supply,Switch)

if Switch >= 1
    Voltage = 5.5; %Volts
    Current = 0.000001; %10 microAmperes
    
    kWUsage2 = (Voltage * Current)/1000;
    
    if kWUsage2 >= Supply
        kWUsage2 = Supply;
    end
    
else
    kWUsage2 = 0;
end
end

== matlab function MCVT_Integration/Sys8 - Interior Environment/Habitat Interior Environment Model/Temperature Sensors/ Resistance Temperature Detector1/R vs T ==
  data: R input; T output
function T = fcn(R)

T = 1.76520388802152e-09 * R^4 - 2.98990866289637e-07 * R^3 + 0.000987249006463873 * R^2 ...
    + 2.36178487086887 * R - 245.924688605077;

end

== matlab function MCVT_Integration/Sys8 - Interior Environment/Habitat Interior Environment Model/Temperature Sensors/ Resistance Temperature Detector1/RTD with Self-heat Error/MATLAB Function ==
  data: I input; R input; U output
function U = fcn(I, R)

U = I * R;     % Operating current = 1mA
               % Voltage output U = I*R

== matlab function MCVT_Integration/Sys8 - Interior Environment/Habitat Interior Environment Model/Temperature Sensors/ Resistance Temperature Detector1/RTD without Self-heat Error/MATLAB Function ==
  data: I input; I output; R input; U output
function [I,U]= fcn(I,R)

U = I * R;     % Operating current = 1mA
               % Voltage output U = I*R

== matlab function MCVT_Integration/Sys8 - Interior Environment/Habitat Interior Environment Model/Temperature Sensors/ Resistance Temperature Detector1/Self-heat Calculation/  T vs R  ==
  data: R output; T input
function R = fcn(T)

if T >= 0
    R = 100*(1+3.9083*10^(-3)*T-5.775*10^(-7)*T^2);
else
    R = 100*(1+3.9083*10^(-3)*T-5.775*10^(-7)*T^2-4.183*10^(-12)*(T-100)*T^3);
end

== matlab function MCVT_Integration/Sys8 - Interior Environment/Habitat Interior Environment Model/Temperature Sensors/ Resistance Temperature Detector1/Switch ON//OFF Control Block/Switch OFF/MATLAB Function1 ==
  data: u input; y output; Time input
function y = fcn(u,Time)

if Time>=0
    u = NaN;
end

y = u;

== matlab function MCVT_Integration/Sys8 - Interior Environment/Habitat Interior Environment Model/Temperature Sensors/ Resistance Temperature Detector1/T vs R ==
  data: R output; T input
function R = fcn(T)

if T >= 0
    R = 100*(1+3.9083*10^(-3)*T-5.775*10^(-7)*T^2);
else
    R = 100*(1+3.9083*10^(-3)*T-5.775*10^(-7)*T^2-4.183*10^(-12)*(T-100)*T^3);
end

== matlab function MCVT_Integration/Sys8 - Interior Environment/Habitat Interior Environment Model/Temperature Sensors/ Resistance Temperature Detector2/Power Consumption ==
  data: Supply input; kWUsage3 output; Switch input
function kWUsage3 = Power_Consumption(Supply,Switch)

if Switch >= 1
    Voltage = 5.5; %Volts
    Current = 0.000001; %10 microAmperes
    
    kWUsage3 = (Voltage * Current)/1000;
    
    if kWUsage3 >= Supply
        kWUsage3 = Supply;
    end
    
else
    kWUsage3 = 0;
end
end

== matlab function MCVT_Integration/Sys 6 - Health Management and Agents/ Agent System/Agent Module/MATLAB Function ==
  data: actionID input; cActionID output
function cActionID = correction(actionID)
    %{
        "Corrects" the actionID of the Agent Module from -9999 to -1
        This is purely for visualization reasons!
    %}
    if actionID < 0
        cActionID = -1;
    else
        cActionID = actionID;
    end
end
